simulink model "VirtualADAPT"  (Simulink 8.9)
settings: Solver=ode45  StartTime=0.0  StopTime=inf  RelTol=1e-3  EnableMultiTasking=on
PostLoadFcn:
  | FaultInjectionGUIHandle = FaultInjectionGUI;
InitFcn:
  | VirtualADAPTDataStructure
undefined symbols (not defined in the file or in its folder): ln
libraries: simulink x363
(part 4 of 26)

== system VirtualADAPT/VirtualADAPTv1/PowerDistribution/ACLoadBank1/RelayEY174 ==
  EP2In0 : Inport
  EP1In0 : Inport  Port=2
  SwitchClose : Inport  Port=3
  ParallelConn : Subsystem
      mask Display: image(imread('0.png','BackgroundColor',[1 1 1]))
  Relay : Subsystem
      mask Display: image(imread('1.png','BackgroundColor',[1 1 1]))
  Sensor : Subsystem
  EP2Out0 : Outport
  EP1Out0 : Outport  Port=2
  RelayState : Outport  Port=3
  Current : Outport  Port=4
  Voltage : Outport  Port=5
  wires:
  Relay/1(y(1)) -> ParallelConn/1(u(1))
  ParallelConn/1(y(1)) -> Relay/1(u(1))
  EP2In0/1 -> ParallelConn/2(u(2))
  ParallelConn/2(y(2)) -> EP2Out0/1
  EP1In0/1 -> Relay/2(u(2))
  Relay/2(y(2)) -> EP1Out0/1
  SwitchClose/1 -> Relay/3(u(3)), ParallelConn/3(u(3))
  Relay/3(O) -> Current/1
  ParallelConn/3(O) -> Voltage/1
  Sensor/1(SensedValue) -> RelayState/1
  Relay/4(State) -> Sensor/1(TrueValue)

== system VirtualADAPT/VirtualADAPTv1/PowerDistribution/ACLoadBank1/RelayEY175 ==
  EP2In0 : Inport
  EP1In0 : Inport  Port=2
  SwitchClose : Inport  Port=3
  ParallelConn : Subsystem
      mask Display: image(imread('0.png','BackgroundColor',[1 1 1]))
  Relay : Subsystem
      mask Display: image(imread('1.png','BackgroundColor',[1 1 1]))
  Sensor : Subsystem
  EP2Out0 : Outport
  EP1Out0 : Outport  Port=2
  RelayState : Outport  Port=3
  Current : Outport  Port=4
  Voltage : Outport  Port=5
  wires:
  Relay/1(y(1)) -> ParallelConn/1(u(1))
  ParallelConn/1(y(1)) -> Relay/1(u(1))
  EP2In0/1 -> ParallelConn/2(u(2))
  ParallelConn/2(y(2)) -> EP2Out0/1
  EP1In0/1 -> Relay/2(u(2))
  Relay/2(y(2)) -> EP1Out0/1
  SwitchClose/1 -> Relay/3(u(3)), ParallelConn/3(u(3))
  Relay/3(O) -> Current/1
  ParallelConn/3(O) -> Voltage/1
  Sensor/1(SensedValue) -> RelayState/1
  Relay/4(State) -> Sensor/1(TrueValue)

== system VirtualADAPT/VirtualADAPTv1/PowerDistribution/ACLoadBank2/L2A ==
  VInIn0 : Inport
  PMP1 : Subsystem
  SensorFT520 : Subsystem
  VInOut0 : Outport
  FT520 : Outport  Port=2
  wires:
  VInIn0/1 -> PMP1/1(InputIn0)
  PMP1/1(InputOut0) -> VInOut0/1
  PMP1/3(Flow) -> SensorFT520/1(TrueValue)
  SensorFT520/1(SensedValue) -> FT520/1

== system VirtualADAPT/VirtualADAPTv1/PowerDistribution/ACLoadBank2/L2B ==
  InputIn0 : Inport
  AmbientTemp : Inport  Port=2
  LGT2 : Subsystem
  SensorTE510 : Subsystem
  InputOut0 : Outport
  TE510 : Outport  Port=2
  Current : Outport  Port=3
  wires:
  InputIn0/1 -> LGT2/1(EPIn0)
  LGT2/1(EPOut0) -> InputOut0/1
  LGT2/4(Temperature) -> SensorTE510/1(TrueValue)
  LGT2/3(Current) -> Current/1
  AmbientTemp/1 -> LGT2/2(AmbientTemp)
  SensorTE510/1(SensedValue) -> TE510/1

== system VirtualADAPT/VirtualADAPTv1/PowerDistribution/ACLoadBank2/L2C ==
  EnergyPortIn0 : Inport
  FAN4 : Subsystem
  EnergyPortOut0 : Outport
  wires:
  EnergyPortIn0/1 -> FAN4/1(EPIn0)
  FAN4/1(EPOut0) -> EnergyPortOut0/1

== system VirtualADAPT/VirtualADAPTv1/PowerDistribution/ACLoadBank2/L2D ==
  EnergyPortIn0 : Inport
  LGT9 : Subsystem
  EnergyPortOut0 : Outport
  wires:
  EnergyPortIn0/1 -> LGT9/1(EPIn0)
  LGT9/1(EPOut0) -> EnergyPortOut0/1

== system VirtualADAPT/VirtualADAPTv1/PowerDistribution/ACLoadBank2/L2E ==
  InputIn0 : Inport
  AmbientTemp : Inport  Port=2
  LGT7 : Subsystem
  SensorLT505 : Subsystem
  SensorTE505 : Subsystem
  SensorTE506 : Subsystem
  SensorTE507 : Subsystem
  InputOut0 : Outport
  TE505 : Outport  Port=2
  LT505 : Outport  Port=3
  TE507 : Outport  Port=4
  TE506 : Outport  Port=5
  wires:
  InputIn0/1 -> LGT7/1(VInIn0)
  LGT7/1(VInOut0) -> InputOut0/1
  LGT7/6(Temperature3) -> SensorTE507/1(TrueValue)
  LGT7/4(Temperature1) -> SensorTE505/1(TrueValue)
  AmbientTemp/1 -> LGT7/2(AmbientTemp)
  LGT7/3(Power) -> SensorLT505/1(Power)
  LGT7/5(Temperature2) -> SensorTE506/1(TrueValue)
  SensorTE507/1(SensedValue) -> TE507/1
  SensorTE506/1(SensedValue) -> TE506/1
  SensorTE505/1(SensedValue) -> TE505/1
  SensorLT505/1(Light) -> LT505/1

== system VirtualADAPT/VirtualADAPTv1/PowerDistribution/ACLoadBank2/L2F ==
  VinIn0 : Inport
  FAN2 : Subsystem
  SensorST516 : Subsystem
  VinOut0 : Outport
  ST516 : Outport  Port=2
  Current : Outport  Port=3
  wires:
  VinIn0/1 -> FAN2/1(InputIn0)
  FAN2/1(InputOut0) -> VinOut0/1
  FAN2/3(Current) -> Current/1
  SensorST516/1(SensedValue) -> ST516/1
  FAN2/2(RotationalVelocity) -> SensorST516/1(TrueValue)

== system VirtualADAPT/VirtualADAPTv1/PowerDistribution/ACLoadBank2/RelayEY270 ==
  EP2In0 : Inport
  EP1In0 : Inport  Port=2
  SwitchClose : Inport  Port=3
  ParallelConn : Subsystem
      mask Display: image(imread('0.png','BackgroundColor',[1 1 1]))
  Relay : Subsystem
      mask Display: image(imread('1.png','BackgroundColor',[1 1 1]))
  Sensor : Subsystem
  EP2Out0 : Outport
  EP1Out0 : Outport  Port=2
  RelayState : Outport  Port=3
  Current : Outport  Port=4
  Voltage : Outport  Port=5
  wires:
  Relay/1(y(1)) -> ParallelConn/1(u(1))
  ParallelConn/1(y(1)) -> Relay/1(u(1))
  EP2In0/1 -> ParallelConn/2(u(2))
  ParallelConn/2(y(2)) -> EP2Out0/1
  EP1In0/1 -> Relay/2(u(2))
  Relay/2(y(2)) -> EP1Out0/1
  SwitchClose/1 -> Relay/3(u(3)), ParallelConn/3(u(3))
  Relay/3(O) -> Current/1
  ParallelConn/3(O) -> Voltage/1
  Sensor/1(SensedValue) -> RelayState/1
  Relay/4(State) -> Sensor/1(TrueValue)

== system VirtualADAPT/VirtualADAPTv1/PowerDistribution/ACLoadBank2/RelayEY271 ==
  EP2In0 : Inport
  EP1In0 : Inport  Port=2
  SwitchClose : Inport  Port=3
  ParallelConn : Subsystem
      mask Display: image(imread('0.png','BackgroundColor',[1 1 1]))
  Relay : Subsystem
      mask Display: image(imread('1.png','BackgroundColor',[1 1 1]))
  Sensor : Subsystem
  EP2Out0 : Outport
  EP1Out0 : Outport  Port=2
  RelayState : Outport  Port=3
  Current : Outport  Port=4
  Voltage : Outport  Port=5
  wires:
  Relay/1(y(1)) -> ParallelConn/1(u(1))
  ParallelConn/1(y(1)) -> Relay/1(u(1))
  EP2In0/1 -> ParallelConn/2(u(2))
  ParallelConn/2(y(2)) -> EP2Out0/1
  EP1In0/1 -> Relay/2(u(2))
  Relay/2(y(2)) -> EP1Out0/1
  SwitchClose/1 -> Relay/3(u(3)), ParallelConn/3(u(3))
  Relay/3(O) -> Current/1
  ParallelConn/3(O) -> Voltage/1
  Sensor/1(SensedValue) -> RelayState/1
  Relay/4(State) -> Sensor/1(TrueValue)

== system VirtualADAPT/VirtualADAPTv1/PowerDistribution/ACLoadBank2/RelayEY272 ==
  EP2In0 : Inport
  EP1In0 : Inport  Port=2
  SwitchClose : Inport  Port=3
  ParallelConn : Subsystem
      mask Display: image(imread('0.png','BackgroundColor',[1 1 1]))
  Relay : Subsystem
      mask Display: image(imread('1.png','BackgroundColor',[1 1 1]))
  Sensor : Subsystem
  EP2Out0 : Outport
  EP1Out0 : Outport  Port=2
  RelayState : Outport  Port=3
  Current : Outport  Port=4
  Voltage : Outport  Port=5
  wires:
  Relay/1(y(1)) -> ParallelConn/1(u(1))
  ParallelConn/1(y(1)) -> Relay/1(u(1))
  EP2In0/1 -> ParallelConn/2(u(2))
  ParallelConn/2(y(2)) -> EP2Out0/1
  EP1In0/1 -> Relay/2(u(2))
  Relay/2(y(2)) -> EP1Out0/1
  SwitchClose/1 -> Relay/3(u(3)), ParallelConn/3(u(3))
  Relay/3(O) -> Current/1
  ParallelConn/3(O) -> Voltage/1
  Sensor/1(SensedValue) -> RelayState/1
  Relay/4(State) -> Sensor/1(TrueValue)

== system VirtualADAPT/VirtualADAPTv1/PowerDistribution/ACLoadBank2/RelayEY273 ==
  EP2In0 : Inport
  EP1In0 : Inport  Port=2
  SwitchClose : Inport  Port=3
  ParallelConn : Subsystem
      mask Display: image(imread('0.png','BackgroundColor',[1 1 1]))
  Relay : Subsystem
      mask Display: image(imread('1.png','BackgroundColor',[1 1 1]))
  Sensor : Subsystem
  EP2Out0 : Outport
  EP1Out0 : Outport  Port=2
  RelayState : Outport  Port=3
  Current : Outport  Port=4
  Voltage : Outport  Port=5
  wires:
  Relay/1(y(1)) -> ParallelConn/1(u(1))
  ParallelConn/1(y(1)) -> Relay/1(u(1))
  EP2In0/1 -> ParallelConn/2(u(2))
  ParallelConn/2(y(2)) -> EP2Out0/1
  EP1In0/1 -> Relay/2(u(2))
  Relay/2(y(2)) -> EP1Out0/1
  SwitchClose/1 -> Relay/3(u(3)), ParallelConn/3(u(3))
  Relay/3(O) -> Current/1
  ParallelConn/3(O) -> Voltage/1
  Sensor/1(SensedValue) -> RelayState/1
  Relay/4(State) -> Sensor/1(TrueValue)

== system VirtualADAPT/VirtualADAPTv1/PowerDistribution/ACLoadBank2/RelayEY274 ==
  EP2In0 : Inport
  EP1In0 : Inport  Port=2
  SwitchClose : Inport  Port=3
  ParallelConn : Subsystem
      mask Display: image(imread('0.png','BackgroundColor',[1 1 1]))
  Relay : Subsystem
      mask Display: image(imread('1.png','BackgroundColor',[1 1 1]))
  Sensor : Subsystem
  EP2Out0 : Outport
  EP1Out0 : Outport  Port=2
  RelayState : Outport  Port=3
  Current : Outport  Port=4
  Voltage : Outport  Port=5
  wires:
  Relay/1(y(1)) -> ParallelConn/1(u(1))
  ParallelConn/1(y(1)) -> Relay/1(u(1))
  EP2In0/1 -> ParallelConn/2(u(2))
  ParallelConn/2(y(2)) -> EP2Out0/1
  EP1In0/1 -> Relay/2(u(2))
  Relay/2(y(2)) -> EP1Out0/1
  SwitchClose/1 -> Relay/3(u(3)), ParallelConn/3(u(3))
  Relay/3(O) -> Current/1
  ParallelConn/3(O) -> Voltage/1
  Sensor/1(SensedValue) -> RelayState/1
  Relay/4(State) -> Sensor/1(TrueValue)

== system VirtualADAPT/VirtualADAPTv1/PowerDistribution/ACLoadBank2/RelayEY275 ==
  EP2In0 : Inport
  EP1In0 : Inport  Port=2
  SwitchClose : Inport  Port=3
  ParallelConn : Subsystem
      mask Display: image(imread('0.png','BackgroundColor',[1 1 1]))
  Relay : Subsystem
      mask Display: image(imread('1.png','BackgroundColor',[1 1 1]))
  Sensor : Subsystem
  EP2Out0 : Outport
  EP1Out0 : Outport  Port=2
  RelayState : Outport  Port=3
  Current : Outport  Port=4
  Voltage : Outport  Port=5
  wires:
  Relay/1(y(1)) -> ParallelConn/1(u(1))
  ParallelConn/1(y(1)) -> Relay/1(u(1))
  EP2In0/1 -> ParallelConn/2(u(2))
  ParallelConn/2(y(2)) -> EP2Out0/1
  EP1In0/1 -> Relay/2(u(2))
  Relay/2(y(2)) -> EP1Out0/1
  SwitchClose/1 -> Relay/3(u(3)), ParallelConn/3(u(3))
  Relay/3(O) -> Current/1
  ParallelConn/3(O) -> Voltage/1
  Sensor/1(SensedValue) -> RelayState/1
  Relay/4(State) -> Sensor/1(TrueValue)

== system VirtualADAPT/VirtualADAPTv1/PowerDistribution/CircuitBreakerEY162/CircuitBreakerConnected ==
  Constant : Constant  Value=Constant
  Fault Inject : Subsystem
  Fault Profile : Constant  Value=FP
  Ramp : simulink/Sources/Ramp  slope=FM  start=FT  X0=Constant
  Step : Step  Time=FT  Before=Constant  After=(1 + FM)*Constant  SampleTime=0
  C : Outport
  wires:
  Fault Inject/1(Out1) -> C/1
  Constant/1 -> Fault Inject/1(Nominal)
  Fault Profile/1 -> Fault Inject/2(Fault Profile)
  Step/1 -> Fault Inject/3(Step)
  Ramp/1 -> Fault Inject/4(Ramp)

== system VirtualADAPT/VirtualADAPTv1/PowerDistribution/CircuitBreakerEY162/CurrentLimit ==
  Constant : Constant  Value=Constant
  Fault Inject : Subsystem
  Fault Profile : Constant  Value=FP
  Ramp : simulink/Sources/Ramp  slope=FM  start=FT  X0=Constant
  Step : Step  Time=FT  Before=Constant  After=(1 + FM)*Constant  SampleTime=0
  C : Outport
  wires:
  Fault Inject/1(Out1) -> C/1
  Constant/1 -> Fault Inject/1(Nominal)
  Fault Profile/1 -> Fault Inject/2(Fault Profile)
  Step/1 -> Fault Inject/3(Step)
  Ramp/1 -> Fault Inject/4(Ramp)

== system VirtualADAPT/VirtualADAPTv1/PowerDistribution/CircuitBreakerEY162/CurrentThrough ==
  u(1) : Inport
  u(2) : Inport  Port=2
  u(3) : Inport  Port=3
  u(4) : Inport  Port=4
  Adder : Sum  Inputs=+  SaturateOnIntegerOverflow=on  InputSameDT=on  OutDataTypeStr=Inherit: Same as first input
  Gain : Gain  SaturateOnIntegerOverflow=on  OutDataTypeStr=Inherit: Same as input  ParamDataTypeStr=Inherit: Same as input
  Mux : Mux
  OffGuard : Fcn  Expr=(u(3) || !u(4))
  OnGuard : Fcn  Expr=(0)
  Product1 : Product  SaturateOnIntegerOverflow=on  RndMeth=Zero  InputSameDT=on  OutDataTypeStr=Inherit: Same as first input
  Product2 : Product  SaturateOnIntegerOverflow=on  RndMeth=Zero  InputSameDT=on  OutDataTypeStr=Inherit: Same as first input
  determineState : Subsystem
  y(1) : Outport
  y(2) : Outport  Port=2
  O : Outport  Port=3
  State : Outport  Port=4
  wires:
  Adder/1 -> Gain/1
  u(3)/1 -> Mux/3
  u(4)/1 -> Mux/4
  Mux/1 -> OnGuard/1, OffGuard/1
  OnGuard/1 -> determineState/1(In1)
  OffGuard/1 -> determineState/2(In2)
  u(1)/1 -> Product1/1
  determineState/1(Out1) -> Product1/2, Product2/2, State/1
  Product1/1 -> O/1, y(2)/1
  Gain/1 -> Product2/1
  Product2/1 -> y(1)/1
  u(2)/1 -> Adder/1

== system VirtualADAPT/VirtualADAPTv1/PowerDistribution/CircuitBreakerEY162/DecisionFunction ==
  u(1) : Inport
  u(2) : Inport  Port=2
  Function : Fcn  Expr=u(1)>u(2)
  Mux : Mux  Inputs=2
  One : Constant
  Switch : Switch  Criteria=u2 ~= 0  SaturateOnIntegerOverflow=on  InputSameDT=on
  Zero : Constant  Value=0
  y(1) : Outport
  wires:
  u(1)/1 -> Mux/1
  u(2)/1 -> Mux/2
  Mux/1 -> Function/1
  Function/1 -> Switch/2
  One/1 -> Switch/1
  Zero/1 -> Switch/3
  Switch/1 -> y(1)/1

== system VirtualADAPT/VirtualADAPTv1/PowerDistribution/CircuitBreakerEY162/Delay ==
  Input : Inport
  C : Subsystem  mask{C=0.1, IC=0, FP=Nominal, FT=0, FM=0}
      mask prompts: C "Value of C:"; IC "Initial Condition:"; FP "Fault Profile:"; FT "Fault Injection Time:"; FM "Fault Magnitude/Slope"
      mask TypeOptions FP: Nominal | Abrupt | Incipient
      mask Display: image(imread('C.png','BackgroundColor',[1 1 1]))
  Function : Subsystem
      mask Display: image(imread('fx.png','BackgroundColor',[1 1 1]))
  OneJunction : Subsystem
      mask Display: image(imread('1.png','BackgroundColor',[1 1 1]))
  R : Subsystem  mask{R=1, FP=Nominal, FT=0, FM=0}
      mask prompts: R "Value of R:"; FP "Fault Profile:"; FT "Fault Injection Time:"; FM "Fault Magnitude/Slope"
      mask TypeOptions FP: Nominal | Abrupt | Incipient
      mask Display: image(imread('R.png','BackgroundColor',[1 1 1]))
  Se : Subsystem  mask{FP=Nominal, FT=0, FM=0}
      mask prompts: FP "Fault Profile:"; FT "Fault Injection Time:"; FM "Fault Magnitude/Slope/Bias/StuckAtValue"
      mask TypeOptions FP: Nominal | Abrupt | Incipient | Bias | StuckAt
      mask Display: image(imread('MSe.png','BackgroundColor',[1 1 1]))
  ZeroJunction : Subsystem
      mask Display: image(imread('0.png','BackgroundColor',[1 1 1]))
  Output : Outport
  wires:
  OneJunction/1(y(1)) -> R/1(In)
  R/1(Out) -> OneJunction/1(u(1))
  ZeroJunction/1(y(1)) -> C/1(F)
  C/1(E) -> ZeroJunction/1(u(1))
  OneJunction/2(y(2)) -> ZeroJunction/2(u(2))
  ZeroJunction/2(y(2)) -> OneJunction/2(u(2))
  Se/1(E) -> OneJunction/3(u(3))
  OneJunction/3(y(3)) -> Se/1(F)
  Function/1(y(1)) -> Se/2(Se)
  Input/1 -> Function/1(u(1))
  ZeroJunction/3(O) -> Output/1

== system VirtualADAPT/VirtualADAPTv1/PowerDistribution/CircuitBreakerEY162/ParallelConn ==
  u(1) : Inport
  u(2) : Inport  Port=2
  u(3) : Inport  Port=3
  u(4) : Inport  Port=4
  Adder : Sum  Inputs=-  SaturateOnIntegerOverflow=on  InputSameDT=on  OutDataTypeStr=Inherit: Same as first input
  Gain : Gain  Gain=-1  SaturateOnIntegerOverflow=on  OutDataTypeStr=Inherit: Same as input  ParamDataTypeStr=Inherit: Same as input
  Mux : Mux
  OffGuard : Fcn  Expr=(u(3) || !u(4))
  OnGuard : Fcn  Expr=(0)
  Product1 : Product  SaturateOnIntegerOverflow=on  RndMeth=Zero  InputSameDT=on  OutDataTypeStr=Inherit: Same as first input
  Product2 : Product  SaturateOnIntegerOverflow=on  RndMeth=Zero  InputSameDT=on  OutDataTypeStr=Inherit: Same as first input
  determineState : Subsystem
  y(1) : Outport
  y(2) : Outport  Port=2
  O : Outport  Port=3
  State : Outport  Port=4
  wires:
  Adder/1 -> Gain/1
  u(3)/1 -> Mux/3
  u(4)/1 -> Mux/4
  Mux/1 -> OnGuard/1, OffGuard/1
  OnGuard/1 -> determineState/1(In1)
  OffGuard/1 -> determineState/2(In2)
  u(1)/1 -> Product1/1
  determineState/1(Out1) -> Product1/2, Product2/2, State/1
  Product1/1 -> O/1, y(2)/1
  Gain/1 -> Product2/1
  Product2/1 -> y(1)/1
  u(2)/1 -> Adder/1

== system VirtualADAPT/VirtualADAPTv1/PowerDistribution/CircuitBreakerEY166/CircuitBreakerConnected ==
  Constant : Constant  Value=Constant
  Fault Inject : Subsystem
  Fault Profile : Constant  Value=FP
  Ramp : simulink/Sources/Ramp  slope=FM  start=FT  X0=Constant
  Step : Step  Time=FT  Before=Constant  After=(1 + FM)*Constant  SampleTime=0
  C : Outport
  wires:
  Fault Inject/1(Out1) -> C/1
  Constant/1 -> Fault Inject/1(Nominal)
  Fault Profile/1 -> Fault Inject/2(Fault Profile)
  Step/1 -> Fault Inject/3(Step)
  Ramp/1 -> Fault Inject/4(Ramp)

== system VirtualADAPT/VirtualADAPTv1/PowerDistribution/CircuitBreakerEY166/CurrentLimit ==
  Constant : Constant  Value=Constant
  Fault Inject : Subsystem
  Fault Profile : Constant  Value=FP
  Ramp : simulink/Sources/Ramp  slope=FM  start=FT  X0=Constant
  Step : Step  Time=FT  Before=Constant  After=(1 + FM)*Constant  SampleTime=0
  C : Outport
  wires:
  Fault Inject/1(Out1) -> C/1
  Constant/1 -> Fault Inject/1(Nominal)
  Fault Profile/1 -> Fault Inject/2(Fault Profile)
  Step/1 -> Fault Inject/3(Step)
  Ramp/1 -> Fault Inject/4(Ramp)

== system VirtualADAPT/VirtualADAPTv1/PowerDistribution/CircuitBreakerEY166/CurrentThrough ==
  u(1) : Inport
  u(2) : Inport  Port=2
  u(3) : Inport  Port=3
  u(4) : Inport  Port=4
  Adder : Sum  Inputs=+  SaturateOnIntegerOverflow=on  InputSameDT=on  OutDataTypeStr=Inherit: Same as first input
  Gain : Gain  SaturateOnIntegerOverflow=on  OutDataTypeStr=Inherit: Same as input  ParamDataTypeStr=Inherit: Same as input
  Mux : Mux
  OffGuard : Fcn  Expr=(u(3) || !u(4))
  OnGuard : Fcn  Expr=(0)
  Product1 : Product  SaturateOnIntegerOverflow=on  RndMeth=Zero  InputSameDT=on  OutDataTypeStr=Inherit: Same as first input
  Product2 : Product  SaturateOnIntegerOverflow=on  RndMeth=Zero  InputSameDT=on  OutDataTypeStr=Inherit: Same as first input
  determineState : Subsystem
  y(1) : Outport
  y(2) : Outport  Port=2
  O : Outport  Port=3
  State : Outport  Port=4
  wires:
  Adder/1 -> Gain/1
  u(3)/1 -> Mux/3
  u(4)/1 -> Mux/4
  Mux/1 -> OnGuard/1, OffGuard/1
  OnGuard/1 -> determineState/1(In1)
  OffGuard/1 -> determineState/2(In2)
  u(1)/1 -> Product1/1
  determineState/1(Out1) -> Product1/2, Product2/2, State/1
  Product1/1 -> O/1, y(2)/1
  Gain/1 -> Product2/1
  Product2/1 -> y(1)/1
  u(2)/1 -> Adder/1

== system VirtualADAPT/VirtualADAPTv1/PowerDistribution/CircuitBreakerEY166/DecisionFunction ==
  u(1) : Inport
  u(2) : Inport  Port=2
  Function : Fcn  Expr=u(1)>u(2)
  Mux : Mux  Inputs=2
  One : Constant
  Switch : Switch  Criteria=u2 ~= 0  SaturateOnIntegerOverflow=on  InputSameDT=on
  Zero : Constant  Value=0
  y(1) : Outport
  wires:
  u(1)/1 -> Mux/1
  u(2)/1 -> Mux/2
  Mux/1 -> Function/1
  Function/1 -> Switch/2
  One/1 -> Switch/1
  Zero/1 -> Switch/3
  Switch/1 -> y(1)/1

== system VirtualADAPT/VirtualADAPTv1/PowerDistribution/CircuitBreakerEY166/Delay ==
  Input : Inport
  C : Subsystem  mask{C=0.1, IC=0, FP=Nominal, FT=0, FM=0}
      mask prompts: C "Value of C:"; IC "Initial Condition:"; FP "Fault Profile:"; FT "Fault Injection Time:"; FM "Fault Magnitude/Slope"
      mask TypeOptions FP: Nominal | Abrupt | Incipient
      mask Display: image(imread('C.png','BackgroundColor',[1 1 1]))
  Function : Subsystem
      mask Display: image(imread('fx.png','BackgroundColor',[1 1 1]))
  OneJunction : Subsystem
      mask Display: image(imread('1.png','BackgroundColor',[1 1 1]))
  R : Subsystem  mask{R=1, FP=Nominal, FT=0, FM=0}
      mask prompts: R "Value of R:"; FP "Fault Profile:"; FT "Fault Injection Time:"; FM "Fault Magnitude/Slope"
      mask TypeOptions FP: Nominal | Abrupt | Incipient
      mask Display: image(imread('R.png','BackgroundColor',[1 1 1]))
  Se : Subsystem  mask{FP=Nominal, FT=0, FM=0}
      mask prompts: FP "Fault Profile:"; FT "Fault Injection Time:"; FM "Fault Magnitude/Slope/Bias/StuckAtValue"
      mask TypeOptions FP: Nominal | Abrupt | Incipient | Bias | StuckAt
      mask Display: image(imread('MSe.png','BackgroundColor',[1 1 1]))
  ZeroJunction : Subsystem
      mask Display: image(imread('0.png','BackgroundColor',[1 1 1]))
  Output : Outport
  wires:
  Se/1(E) -> OneJunction/1(u(1))
  OneJunction/1(y(1)) -> Se/1(F)
  OneJunction/2(y(2)) -> ZeroJunction/1(u(1))
  ZeroJunction/1(y(1)) -> OneJunction/2(u(2))
  ZeroJunction/2(y(2)) -> C/1(F)
  C/1(E) -> ZeroJunction/2(u(2))
  OneJunction/3(y(3)) -> R/1(In)
  R/1(Out) -> OneJunction/3(u(3))
  Function/1(y(1)) -> Se/2(Se)
  Input/1 -> Function/1(u(1))
  ZeroJunction/3(O) -> Output/1

== system VirtualADAPT/VirtualADAPTv1/PowerDistribution/CircuitBreakerEY166/ParallelConn ==
  u(1) : Inport
  u(2) : Inport  Port=2
  u(3) : Inport  Port=3
  u(4) : Inport  Port=4
  u(5) : Inport  Port=5
  u(6) : Inport  Port=6
  u(7) : Inport  Port=7
  u(8) : Inport  Port=8
  u(9) : Inport  Port=9
  Adder : Sum  Inputs=------  SaturateOnIntegerOverflow=on  InputSameDT=on  OutDataTypeStr=Inherit: Same as first input
  Gain : Gain  Gain=-1  SaturateOnIntegerOverflow=on  OutDataTypeStr=Inherit: Same as input  ParamDataTypeStr=Inherit: Same as input
  Mux : Mux  Inputs=9
  OffGuard : Fcn  Expr=(u(8) || !u(9))
  OnGuard : Fcn  Expr=(0)
  Product1 : Product  SaturateOnIntegerOverflow=on  RndMeth=Zero  InputSameDT=on  OutDataTypeStr=Inherit: Same as first input
  Product2 : Product  SaturateOnIntegerOverflow=on  RndMeth=Zero  InputSameDT=on  OutDataTypeStr=Inherit: Same as first input
  determineState : Subsystem
  y(1) : Outport
  y(2) : Outport  Port=2
  y(3) : Outport  Port=3
  y(4) : Outport  Port=4
  y(5) : Outport  Port=5
  y(6) : Outport  Port=6
  y(7) : Outport  Port=7
  O : Outport  Port=8
  State : Outport  Port=9
  wires:
  Adder/1 -> Gain/1
  u(8)/1 -> Mux/8
  u(9)/1 -> Mux/9
  Mux/1 -> OnGuard/1, OffGuard/1
  OnGuard/1 -> determineState/1(In1)
  OffGuard/1 -> determineState/2(In2)
  u(1)/1 -> Product1/1
  determineState/1(Out1) -> Product1/2, Product2/2, State/1
  Product1/1 -> O/1, y(2)/1, y(3)/1, y(4)/1, y(5)/1, y(6)/1, y(7)/1
  Gain/1 -> Product2/1
  Product2/1 -> y(1)/1
  u(2)/1 -> Adder/1
  u(3)/1 -> Adder/2
  u(4)/1 -> Adder/3
  u(5)/1 -> Adder/4
  u(6)/1 -> Adder/5
  u(7)/1 -> Adder/6

== system VirtualADAPT/VirtualADAPTv1/PowerDistribution/CircuitBreakerEY180/C ==
  F : Inport
  Capacitance : Constant  Value=C
  Fault Inject : Subsystem
  Fault Profile : Constant  Value=FP
  InitialCondition : Constant  Value=IC
  Integrator : Integrator  InitialConditionSource=external
  Product1 : Product  SaturateOnIntegerOverflow=on  RndMeth=Zero  InputSameDT=on  OutDataTypeStr=Inherit: Same as first input
  Product2 : Product  SaturateOnIntegerOverflow=on  RndMeth=Zero  InputSameDT=on  OutDataTypeStr=Inherit: Same as first input
  Ramp : simulink/Sources/Ramp  slope=FM  start=FT  X0=C
  Reciprocal : Math  Operator=reciprocal  SaturateOnIntegerOverflow=on
  Step : Step  Time=FT  Before=C  After=(1 + FM)*C  SampleTime=0
  E : Outport
  C : Outport  Port=2
  q : Outport  Port=3
  wires:
  F/1 -> Integrator/1
  InitialCondition/1 -> Product1/1
  Capacitance/1 -> Fault Inject/1(Nominal), Product1/2
  Product1/1 -> Integrator/2
  Integrator/1 -> Product2/1, q/1
  Fault Inject/1(Out1) -> Reciprocal/1, C/1
  Reciprocal/1 -> Product2/2
  Fault Profile/1 -> Fault Inject/2(Fault Profile)
  Step/1 -> Fault Inject/3(Step)
  Ramp/1 -> Fault Inject/4(Ramp)
  Product2/1 -> E/1

== system VirtualADAPT/VirtualADAPTv1/PowerDistribution/CircuitBreakerEY180/CircuitBreakerConnected ==
  Constant : Constant  Value=Constant
  Fault Inject : Subsystem
  Fault Profile : Constant  Value=FP
  Ramp : simulink/Sources/Ramp  slope=FM  start=FT  X0=Constant
  Step : Step  Time=FT  Before=Constant  After=(1 + FM)*Constant  SampleTime=0
  C : Outport
  wires:
  Fault Inject/1(Out1) -> C/1
  Constant/1 -> Fault Inject/1(Nominal)
  Fault Profile/1 -> Fault Inject/2(Fault Profile)
  Step/1 -> Fault Inject/3(Step)
  Ramp/1 -> Fault Inject/4(Ramp)

== system VirtualADAPT/VirtualADAPTv1/PowerDistribution/CircuitBreakerEY180/CurrentLimit ==
  Constant : Constant  Value=Constant
  Fault Inject : Subsystem
  Fault Profile : Constant  Value=FP
  Ramp : simulink/Sources/Ramp  slope=FM  start=FT  X0=Constant
  Step : Step  Time=FT  Before=Constant  After=(1 + FM)*Constant  SampleTime=0
  C : Outport
  wires:
  Fault Inject/1(Out1) -> C/1
  Constant/1 -> Fault Inject/1(Nominal)
  Fault Profile/1 -> Fault Inject/2(Fault Profile)
  Step/1 -> Fault Inject/3(Step)
  Ramp/1 -> Fault Inject/4(Ramp)

== system VirtualADAPT/VirtualADAPTv1/PowerDistribution/CircuitBreakerEY180/CurrentThrough ==
  u(1) : Inport
  u(2) : Inport  Port=2
  u(3) : Inport  Port=3
  u(4) : Inport  Port=4
  u(5) : Inport  Port=5
  Adder : Sum  Inputs=-+  SaturateOnIntegerOverflow=on  InputSameDT=on  OutDataTypeStr=Inherit: Same as first input
  Gain : Gain  SaturateOnIntegerOverflow=on  OutDataTypeStr=Inherit: Same as input  ParamDataTypeStr=Inherit: Same as input
  Mux : Mux  Inputs=5
  OffGuard : Fcn  Expr=(u(4) || !u(5))
  OnGuard : Fcn  Expr=(0)
  Product1 : Product  SaturateOnIntegerOverflow=on  RndMeth=Zero  InputSameDT=on  OutDataTypeStr=Inherit: Same as first input
  Product2 : Product  SaturateOnIntegerOverflow=on  RndMeth=Zero  InputSameDT=on  OutDataTypeStr=Inherit: Same as first input
  determineState : Subsystem
  y(1) : Outport
  y(2) : Outport  Port=2
  y(3) : Outport  Port=3
  O : Outport  Port=4
  State : Outport  Port=5
  wires:
  Adder/1 -> Gain/1
  u(4)/1 -> Mux/4
  u(5)/1 -> Mux/5
  Mux/1 -> OnGuard/1, OffGuard/1
  OnGuard/1 -> determineState/1(In1)
  OffGuard/1 -> determineState/2(In2)
  u(2)/1 -> Product1/1
  determineState/1(Out1) -> Product1/2, Product2/2, State/1
  Product1/1 -> O/1, y(1)/1, y(3)/1
  Gain/1 -> Product2/1
  u(1)/1 -> Adder/1
  Product2/1 -> y(2)/1
  u(3)/1 -> Adder/2

== system VirtualADAPT/VirtualADAPTv1/PowerDistribution/CircuitBreakerEY180/DecisionFunction ==
  u(1) : Inport
  u(2) : Inport  Port=2
  Function : Fcn  Expr=u(2)>u(1)
  Mux : Mux  Inputs=2
  One : Constant
  Switch : Switch  Criteria=u2 ~= 0  SaturateOnIntegerOverflow=on  InputSameDT=on
  Zero : Constant  Value=0
  y(1) : Outport
  wires:
  u(1)/1 -> Mux/1
  u(2)/1 -> Mux/2
  Mux/1 -> Function/1
  Function/1 -> Switch/2
  One/1 -> Switch/1
  Zero/1 -> Switch/3
  Switch/1 -> y(1)/1

== system VirtualADAPT/VirtualADAPTv1/PowerDistribution/CircuitBreakerEY180/Delay ==
  Input : Inport
  C : Subsystem  mask{C=0.8, IC=0, FP=Nominal, FT=0, FM=0}
      mask prompts: C "Value of C:"; IC "Initial Condition:"; FP "Fault Profile:"; FT "Fault Injection Time:"; FM "Fault Magnitude/Slope"
      mask TypeOptions FP: Nominal | Abrupt | Incipient
      mask Display: image(imread('C.png','BackgroundColor',[1 1 1]))
  Function : Subsystem
      mask Display: image(imread('fx.png','BackgroundColor',[1 1 1]))
  OneJunction : Subsystem
      mask Display: image(imread('1.png','BackgroundColor',[1 1 1]))
  R : Subsystem  mask{R=1, FP=Nominal, FT=0, FM=0}
      mask prompts: R "Value of R:"; FP "Fault Profile:"; FT "Fault Injection Time:"; FM "Fault Magnitude/Slope"
      mask TypeOptions FP: Nominal | Abrupt | Incipient
      mask Display: image(imread('R.png','BackgroundColor',[1 1 1]))
  Se : Subsystem  mask{FP=Nominal, FT=0, FM=0}
      mask prompts: FP "Fault Profile:"; FT "Fault Injection Time:"; FM "Fault Magnitude/Slope/Bias/StuckAtValue"
      mask TypeOptions FP: Nominal | Abrupt | Incipient | Bias | StuckAt
      mask Display: image(imread('MSe.png','BackgroundColor',[1 1 1]))
  ZeroJunction : Subsystem
      mask Display: image(imread('0.png','BackgroundColor',[1 1 1]))
  Output : Outport
  wires:
  Se/1(E) -> OneJunction/1(u(1))
  OneJunction/1(y(1)) -> Se/1(F)
  OneJunction/2(y(2)) -> ZeroJunction/1(u(1))
  ZeroJunction/1(y(1)) -> OneJunction/2(u(2))
  OneJunction/3(y(3)) -> R/1(In)
  R/1(Out) -> OneJunction/3(u(3))
  ZeroJunction/2(y(2)) -> C/1(F)
  C/1(E) -> ZeroJunction/2(u(2))
  Function/1(y(1)) -> Se/2(Se)
  Input/1 -> Function/1(u(1))
  ZeroJunction/3(O) -> Output/1

== system VirtualADAPT/VirtualADAPTv1/PowerDistribution/CircuitBreakerEY180/ParallelConn ==
  u(1) : Inport
  u(2) : Inport  Port=2
  u(3) : Inport  Port=3
  u(4) : Inport  Port=4
  u(5) : Inport  Port=5
  Adder : Sum  Inputs=--  SaturateOnIntegerOverflow=on  InputSameDT=on  OutDataTypeStr=Inherit: Same as first input
  Gain : Gain  Gain=-1  SaturateOnIntegerOverflow=on  OutDataTypeStr=Inherit: Same as input  ParamDataTypeStr=Inherit: Same as input
  Mux : Mux  Inputs=5
  OffGuard : Fcn  Expr=(u(4) || !u(5))
  OnGuard : Fcn  Expr=(0)
  Product1 : Product  SaturateOnIntegerOverflow=on  RndMeth=Zero  InputSameDT=on  OutDataTypeStr=Inherit: Same as first input
  Product2 : Product  SaturateOnIntegerOverflow=on  RndMeth=Zero  InputSameDT=on  OutDataTypeStr=Inherit: Same as first input
  determineState : Subsystem
  y(1) : Outport
  y(2) : Outport  Port=2
  y(3) : Outport  Port=3
  O : Outport  Port=4
  State : Outport  Port=5
  wires:
  Adder/1 -> Gain/1
  u(4)/1 -> Mux/4
  u(5)/1 -> Mux/5
  Mux/1 -> OnGuard/1, OffGuard/1
  OnGuard/1 -> determineState/1(In1)
  OffGuard/1 -> determineState/2(In2)
  u(1)/1 -> Product1/1
  determineState/1(Out1) -> Product1/2, Product2/2, State/1
  Product1/1 -> O/1, y(2)/1, y(3)/1
  Gain/1 -> Product2/1
  Product2/1 -> y(1)/1
  u(2)/1 -> Adder/1
  u(3)/1 -> Adder/2

== system VirtualADAPT/VirtualADAPTv1/PowerDistribution/CircuitBreakerEY180/R ==
  In : Inport
  Bond : Constant  Value=2
  Computing R : Subsystem
  Fault Profile : Constant  Value=FP
  JuncDB : Constant  Value=3
  Junction Type : Constant  Value=0
  Ramp : simulink/Sources/Ramp  slope=FM  start=FT  X0=R
  Resistor Value : Constant  Value=R
  Step : Step  Time=FT  Before=R  After=(1 + FM)*R  SampleTime=0
  Out : Outport
  R : Outport  Port=2
  wires:
  Computing R/1(Out1) -> Out/1
  In/1 -> Computing R/1(In1)
  Resistor Value/1 -> Computing R/2(RNominal)
  Fault Profile/1 -> Computing R/3(Fault Profile)
  Step/1 -> Computing R/4(Step)
  Ramp/1 -> Computing R/5(Ramp)
  JuncDB/1 -> Computing R/6(JuncDB)
  Bond/1 -> Computing R/7(Bond)
  Junction Type/1 -> Computing R/8(Junction Type)
  Computing R/2(Out2) -> R/1

== system VirtualADAPT/VirtualADAPTv1/PowerDistribution/CircuitBreakerEY180/ZeroJunc ==
  u(1) : Inport
  u(2) : Inport  Port=2
  u(3) : Inport  Port=3
  Adder : Sum  Inputs=+-  SaturateOnIntegerOverflow=on  InputSameDT=on  OutDataTypeStr=Inherit: Same as first input
  Gain : Gain  SaturateOnIntegerOverflow=on  OutDataTypeStr=Inherit: Same as input  ParamDataTypeStr=Inherit: Same as input
  y(1) : Outport
  y(2) : Outport  Port=2
  y(3) : Outport  Port=3
  O : Outport  Port=4
  wires:
  Adder/1 -> Gain/1
  u(3)/1 -> y(1)/1, y(2)/1, O/1
  u(1)/1 -> Adder/1
  u(2)/1 -> Adder/2
  Gain/1 -> y(3)/1

== system VirtualADAPT/VirtualADAPTv1/PowerDistribution/CircuitBreakerEY262/CircuitBreakerConnected ==
  Constant : Constant  Value=Constant
  Fault Inject : Subsystem
  Fault Profile : Constant  Value=FP
  Ramp : simulink/Sources/Ramp  slope=FM  start=FT  X0=Constant
  Step : Step  Time=FT  Before=Constant  After=(1 + FM)*Constant  SampleTime=0
  C : Outport
  wires:
  Fault Inject/1(Out1) -> C/1
  Constant/1 -> Fault Inject/1(Nominal)
  Fault Profile/1 -> Fault Inject/2(Fault Profile)
  Step/1 -> Fault Inject/3(Step)
  Ramp/1 -> Fault Inject/4(Ramp)

== system VirtualADAPT/VirtualADAPTv1/PowerDistribution/CircuitBreakerEY262/CurrentLimit ==
  Constant : Constant  Value=Constant
  Fault Inject : Subsystem
  Fault Profile : Constant  Value=FP
  Ramp : simulink/Sources/Ramp  slope=FM  start=FT  X0=Constant
  Step : Step  Time=FT  Before=Constant  After=(1 + FM)*Constant  SampleTime=0
  C : Outport
  wires:
  Fault Inject/1(Out1) -> C/1
  Constant/1 -> Fault Inject/1(Nominal)
  Fault Profile/1 -> Fault Inject/2(Fault Profile)
  Step/1 -> Fault Inject/3(Step)
  Ramp/1 -> Fault Inject/4(Ramp)

== system VirtualADAPT/VirtualADAPTv1/PowerDistribution/CircuitBreakerEY262/CurrentThrough ==
  u(1) : Inport
  u(2) : Inport  Port=2
  u(3) : Inport  Port=3
  u(4) : Inport  Port=4
  Adder : Sum  Inputs=+  SaturateOnIntegerOverflow=on  InputSameDT=on  OutDataTypeStr=Inherit: Same as first input
  Gain : Gain  SaturateOnIntegerOverflow=on  OutDataTypeStr=Inherit: Same as input  ParamDataTypeStr=Inherit: Same as input
  Mux : Mux
  OffGuard : Fcn  Expr=(u(3) || !u(4))
  OnGuard : Fcn  Expr=(0)
  Product1 : Product  SaturateOnIntegerOverflow=on  RndMeth=Zero  InputSameDT=on  OutDataTypeStr=Inherit: Same as first input
  Product2 : Product  SaturateOnIntegerOverflow=on  RndMeth=Zero  InputSameDT=on  OutDataTypeStr=Inherit: Same as first input
  determineState : Subsystem
  y(1) : Outport
  y(2) : Outport  Port=2
  O : Outport  Port=3
  State : Outport  Port=4
  wires:
  Adder/1 -> Gain/1
  u(3)/1 -> Mux/3
  u(4)/1 -> Mux/4
  Mux/1 -> OnGuard/1, OffGuard/1
  OnGuard/1 -> determineState/1(In1)
  OffGuard/1 -> determineState/2(In2)
  u(1)/1 -> Product1/1
  determineState/1(Out1) -> Product1/2, Product2/2, State/1
  Product1/1 -> O/1, y(2)/1
  Gain/1 -> Product2/1
  Product2/1 -> y(1)/1
  u(2)/1 -> Adder/1

== system VirtualADAPT/VirtualADAPTv1/PowerDistribution/CircuitBreakerEY262/DecisionFunction ==
  u(1) : Inport
  u(2) : Inport  Port=2
  Function : Fcn  Expr=u(2)>u(1)
  Mux : Mux  Inputs=2
  One : Constant
  Switch : Switch  Criteria=u2 ~= 0  SaturateOnIntegerOverflow=on  InputSameDT=on
  Zero : Constant  Value=0
  y(1) : Outport
  wires:
  u(1)/1 -> Mux/1
  u(2)/1 -> Mux/2
  Mux/1 -> Function/1
  Function/1 -> Switch/2
  One/1 -> Switch/1
  Zero/1 -> Switch/3
  Switch/1 -> y(1)/1

== system VirtualADAPT/VirtualADAPTv1/PowerDistribution/CircuitBreakerEY262/Delay ==
  Input : Inport
  C : Subsystem  mask{C=0.1, IC=0, FP=Nominal, FT=0, FM=0}
      mask prompts: C "Value of C:"; IC "Initial Condition:"; FP "Fault Profile:"; FT "Fault Injection Time:"; FM "Fault Magnitude/Slope"
      mask TypeOptions FP: Nominal | Abrupt | Incipient
      mask Display: image(imread('C.png','BackgroundColor',[1 1 1]))
  Function : Subsystem
      mask Display: image(imread('fx.png','BackgroundColor',[1 1 1]))
  OneJunction : Subsystem
      mask Display: image(imread('1.png','BackgroundColor',[1 1 1]))
  R : Subsystem  mask{R=1, FP=Nominal, FT=0, FM=0}
      mask prompts: R "Value of R:"; FP "Fault Profile:"; FT "Fault Injection Time:"; FM "Fault Magnitude/Slope"
      mask TypeOptions FP: Nominal | Abrupt | Incipient
      mask Display: image(imread('R.png','BackgroundColor',[1 1 1]))
  Se : Subsystem  mask{FP=Nominal, FT=0, FM=0}
      mask prompts: FP "Fault Profile:"; FT "Fault Injection Time:"; FM "Fault Magnitude/Slope/Bias/StuckAtValue"
      mask TypeOptions FP: Nominal | Abrupt | Incipient | Bias | StuckAt
      mask Display: image(imread('MSe.png','BackgroundColor',[1 1 1]))
  ZeroJunction : Subsystem
      mask Display: image(imread('0.png','BackgroundColor',[1 1 1]))
  Output : Outport
  wires:
  OneJunction/1(y(1)) -> ZeroJunction/1(u(1))
  ZeroJunction/1(y(1)) -> OneJunction/1(u(1))
  OneJunction/2(y(2)) -> R/1(In)
  R/1(Out) -> OneJunction/2(u(2))
  ZeroJunction/2(y(2)) -> C/1(F)
  C/1(E) -> ZeroJunction/2(u(2))
  Se/1(E) -> OneJunction/3(u(3))
  OneJunction/3(y(3)) -> Se/1(F)
  Function/1(y(1)) -> Se/2(Se)
  Input/1 -> Function/1(u(1))
  ZeroJunction/3(O) -> Output/1

== system VirtualADAPT/VirtualADAPTv1/PowerDistribution/CircuitBreakerEY262/ParallelConn ==
  u(1) : Inport
  u(2) : Inport  Port=2
  u(3) : Inport  Port=3
  u(4) : Inport  Port=4
  Adder : Sum  Inputs=-  SaturateOnIntegerOverflow=on  InputSameDT=on  OutDataTypeStr=Inherit: Same as first input
  Gain : Gain  Gain=-1  SaturateOnIntegerOverflow=on  OutDataTypeStr=Inherit: Same as input  ParamDataTypeStr=Inherit: Same as input
  Mux : Mux
  OffGuard : Fcn  Expr=(u(3) || !u(4))
  OnGuard : Fcn  Expr=(0)
  Product1 : Product  SaturateOnIntegerOverflow=on  RndMeth=Zero  InputSameDT=on  OutDataTypeStr=Inherit: Same as first input
  Product2 : Product  SaturateOnIntegerOverflow=on  RndMeth=Zero  InputSameDT=on  OutDataTypeStr=Inherit: Same as first input
  determineState : Subsystem
  y(1) : Outport
  y(2) : Outport  Port=2
  O : Outport  Port=3
  State : Outport  Port=4
  wires:
  Adder/1 -> Gain/1
  u(3)/1 -> Mux/3
  u(4)/1 -> Mux/4
  Mux/1 -> OnGuard/1, OffGuard/1
  OnGuard/1 -> determineState/1(In1)
  OffGuard/1 -> determineState/2(In2)
  u(1)/1 -> Product1/1
  determineState/1(Out1) -> Product1/2, Product2/2, State/1
  Product1/1 -> O/1, y(2)/1
  Gain/1 -> Product2/1
  Product2/1 -> y(1)/1
  u(2)/1 -> Adder/1

== system VirtualADAPT/VirtualADAPTv1/PowerDistribution/CircuitBreakerEY266/CircuitBreakerConnected ==
  Constant : Constant  Value=Constant
  Fault Inject : Subsystem
  Fault Profile : Constant  Value=FP
  Ramp : simulink/Sources/Ramp  slope=FM  start=FT  X0=Constant
  Step : Step  Time=FT  Before=Constant  After=(1 + FM)*Constant  SampleTime=0
  C : Outport
  wires:
  Fault Inject/1(Out1) -> C/1
  Constant/1 -> Fault Inject/1(Nominal)
  Fault Profile/1 -> Fault Inject/2(Fault Profile)
  Step/1 -> Fault Inject/3(Step)
  Ramp/1 -> Fault Inject/4(Ramp)

== system VirtualADAPT/VirtualADAPTv1/PowerDistribution/CircuitBreakerEY266/CurrentLimit ==
  Constant : Constant  Value=Constant
  Fault Inject : Subsystem
  Fault Profile : Constant  Value=FP
  Ramp : simulink/Sources/Ramp  slope=FM  start=FT  X0=Constant
  Step : Step  Time=FT  Before=Constant  After=(1 + FM)*Constant  SampleTime=0
  C : Outport
  wires:
  Fault Inject/1(Out1) -> C/1
  Constant/1 -> Fault Inject/1(Nominal)
  Fault Profile/1 -> Fault Inject/2(Fault Profile)
  Step/1 -> Fault Inject/3(Step)
  Ramp/1 -> Fault Inject/4(Ramp)

== system VirtualADAPT/VirtualADAPTv1/PowerDistribution/CircuitBreakerEY266/CurrentThrough ==
  u(1) : Inport
  u(2) : Inport  Port=2
  u(3) : Inport  Port=3
  u(4) : Inport  Port=4
  Adder : Sum  Inputs=+  SaturateOnIntegerOverflow=on  InputSameDT=on  OutDataTypeStr=Inherit: Same as first input
  Gain : Gain  SaturateOnIntegerOverflow=on  OutDataTypeStr=Inherit: Same as input  ParamDataTypeStr=Inherit: Same as input
  Mux : Mux
  OffGuard : Fcn  Expr=(u(3) || !u(4))
  OnGuard : Fcn  Expr=(0)
  Product1 : Product  SaturateOnIntegerOverflow=on  RndMeth=Zero  InputSameDT=on  OutDataTypeStr=Inherit: Same as first input
  Product2 : Product  SaturateOnIntegerOverflow=on  RndMeth=Zero  InputSameDT=on  OutDataTypeStr=Inherit: Same as first input
  determineState : Subsystem
  y(1) : Outport
  y(2) : Outport  Port=2
  O : Outport  Port=3
  State : Outport  Port=4
  wires:
  Adder/1 -> Gain/1
  u(3)/1 -> Mux/3
  u(4)/1 -> Mux/4
  Mux/1 -> OnGuard/1, OffGuard/1
  OnGuard/1 -> determineState/1(In1)
  OffGuard/1 -> determineState/2(In2)
  u(1)/1 -> Product1/1
  determineState/1(Out1) -> Product1/2, Product2/2, State/1
  Product1/1 -> O/1, y(2)/1
  Gain/1 -> Product2/1
  Product2/1 -> y(1)/1
  u(2)/1 -> Adder/1

== system VirtualADAPT/VirtualADAPTv1/PowerDistribution/CircuitBreakerEY266/DecisionFunction ==
  u(1) : Inport
  u(2) : Inport  Port=2
  Function : Fcn  Expr=u(1)>u(2)
  Mux : Mux  Inputs=2
  One : Constant
  Switch : Switch  Criteria=u2 ~= 0  SaturateOnIntegerOverflow=on  InputSameDT=on
  Zero : Constant  Value=0
  y(1) : Outport
  wires:
  u(1)/1 -> Mux/1
  u(2)/1 -> Mux/2
  Mux/1 -> Function/1
  Function/1 -> Switch/2
  One/1 -> Switch/1
  Zero/1 -> Switch/3
  Switch/1 -> y(1)/1

== system VirtualADAPT/VirtualADAPTv1/PowerDistribution/CircuitBreakerEY266/Delay ==
  Input : Inport
  C : Subsystem  mask{C=0.1, IC=0, FP=Nominal, FT=0, FM=0}
      mask prompts: C "Value of C:"; IC "Initial Condition:"; FP "Fault Profile:"; FT "Fault Injection Time:"; FM "Fault Magnitude/Slope"
      mask TypeOptions FP: Nominal | Abrupt | Incipient
      mask Display: image(imread('C.png','BackgroundColor',[1 1 1]))
  Function : Subsystem
      mask Display: image(imread('fx.png','BackgroundColor',[1 1 1]))
  OneJunction : Subsystem
      mask Display: image(imread('1.png','BackgroundColor',[1 1 1]))
  R : Subsystem  mask{R=1, FP=Nominal, FT=0, FM=0}
      mask prompts: R "Value of R:"; FP "Fault Profile:"; FT "Fault Injection Time:"; FM "Fault Magnitude/Slope"
      mask TypeOptions FP: Nominal | Abrupt | Incipient
      mask Display: image(imread('R.png','BackgroundColor',[1 1 1]))
  Se : Subsystem  mask{FP=Nominal, FT=0, FM=0}
      mask prompts: FP "Fault Profile:"; FT "Fault Injection Time:"; FM "Fault Magnitude/Slope/Bias/StuckAtValue"
      mask TypeOptions FP: Nominal | Abrupt | Incipient | Bias | StuckAt
      mask Display: image(imread('MSe.png','BackgroundColor',[1 1 1]))
  ZeroJunction : Subsystem
      mask Display: image(imread('0.png','BackgroundColor',[1 1 1]))
  Output : Outport
  wires:
  Se/1(E) -> OneJunction/1(u(1))
  OneJunction/1(y(1)) -> Se/1(F)
  OneJunction/2(y(2)) -> ZeroJunction/1(u(1))
  ZeroJunction/1(y(1)) -> OneJunction/2(u(2))
  ZeroJunction/2(y(2)) -> C/1(F)
  C/1(E) -> ZeroJunction/2(u(2))
  OneJunction/3(y(3)) -> R/1(In)
  R/1(Out) -> OneJunction/3(u(3))
  Function/1(y(1)) -> Se/2(Se)
  Input/1 -> Function/1(u(1))
  ZeroJunction/3(O) -> Output/1

== system VirtualADAPT/VirtualADAPTv1/PowerDistribution/CircuitBreakerEY266/ParallelConn ==
  u(1) : Inport
  u(2) : Inport  Port=2
  u(3) : Inport  Port=3
  u(4) : Inport  Port=4
  u(5) : Inport  Port=5
  u(6) : Inport  Port=6
  u(7) : Inport  Port=7
  u(8) : Inport  Port=8
  u(9) : Inport  Port=9
  Adder : Sum  Inputs=------  SaturateOnIntegerOverflow=on  InputSameDT=on  OutDataTypeStr=Inherit: Same as first input
  Gain : Gain  Gain=-1  SaturateOnIntegerOverflow=on  OutDataTypeStr=Inherit: Same as input  ParamDataTypeStr=Inherit: Same as input
  Mux : Mux  Inputs=9
  OffGuard : Fcn  Expr=(u(8) || !u(9))
  OnGuard : Fcn  Expr=(0)
  Product1 : Product  SaturateOnIntegerOverflow=on  RndMeth=Zero  InputSameDT=on  OutDataTypeStr=Inherit: Same as first input
  Product2 : Product  SaturateOnIntegerOverflow=on  RndMeth=Zero  InputSameDT=on  OutDataTypeStr=Inherit: Same as first input
  determineState : Subsystem
  y(1) : Outport
  y(2) : Outport  Port=2
  y(3) : Outport  Port=3
  y(4) : Outport  Port=4
  y(5) : Outport  Port=5
  y(6) : Outport  Port=6
  y(7) : Outport  Port=7
  O : Outport  Port=8
  State : Outport  Port=9
  wires:
  Adder/1 -> Gain/1
  u(8)/1 -> Mux/8
  u(9)/1 -> Mux/9
  Mux/1 -> OnGuard/1, OffGuard/1
  OnGuard/1 -> determineState/1(In1)
  OffGuard/1 -> determineState/2(In2)
  u(1)/1 -> Product1/1
  determineState/1(Out1) -> Product1/2, Product2/2, State/1
  Product1/1 -> O/1, y(2)/1, y(3)/1, y(4)/1, y(5)/1, y(6)/1, y(7)/1
  Gain/1 -> Product2/1
  Product2/1 -> y(1)/1
  u(2)/1 -> Adder/1
  u(3)/1 -> Adder/2
  u(4)/1 -> Adder/3
  u(5)/1 -> Adder/4
  u(6)/1 -> Adder/5
  u(7)/1 -> Adder/6

== system VirtualADAPT/VirtualADAPTv1/PowerDistribution/CircuitBreakerEY280/C ==
  F : Inport
  Capacitance : Constant  Value=C
  Fault Inject : Subsystem
  Fault Profile : Constant  Value=FP
  InitialCondition : Constant  Value=IC
  Integrator : Integrator  InitialConditionSource=external
  Product1 : Product  SaturateOnIntegerOverflow=on  RndMeth=Zero  InputSameDT=on  OutDataTypeStr=Inherit: Same as first input
  Product2 : Product  SaturateOnIntegerOverflow=on  RndMeth=Zero  InputSameDT=on  OutDataTypeStr=Inherit: Same as first input
  Ramp : simulink/Sources/Ramp  slope=FM  start=FT  X0=C
  Reciprocal : Math  Operator=reciprocal  SaturateOnIntegerOverflow=on
  Step : Step  Time=FT  Before=C  After=(1 + FM)*C  SampleTime=0
  E : Outport
  C : Outport  Port=2
  q : Outport  Port=3
  wires:
  F/1 -> Integrator/1
  InitialCondition/1 -> Product1/1
  Capacitance/1 -> Fault Inject/1(Nominal), Product1/2
  Product1/1 -> Integrator/2
  Integrator/1 -> Product2/1, q/1
  Fault Inject/1(Out1) -> Reciprocal/1, C/1
  Reciprocal/1 -> Product2/2
  Fault Profile/1 -> Fault Inject/2(Fault Profile)
  Step/1 -> Fault Inject/3(Step)
  Ramp/1 -> Fault Inject/4(Ramp)
  Product2/1 -> E/1

== system VirtualADAPT/VirtualADAPTv1/PowerDistribution/CircuitBreakerEY280/CircuitBreakerConnected ==
  Constant : Constant  Value=Constant
  Fault Inject : Subsystem
  Fault Profile : Constant  Value=FP
  Ramp : simulink/Sources/Ramp  slope=FM  start=FT  X0=Constant
  Step : Step  Time=FT  Before=Constant  After=(1 + FM)*Constant  SampleTime=0
  C : Outport
  wires:
  Fault Inject/1(Out1) -> C/1
  Constant/1 -> Fault Inject/1(Nominal)
  Fault Profile/1 -> Fault Inject/2(Fault Profile)
  Step/1 -> Fault Inject/3(Step)
  Ramp/1 -> Fault Inject/4(Ramp)

== system VirtualADAPT/VirtualADAPTv1/PowerDistribution/CircuitBreakerEY280/CurrentLimit ==
  Constant : Constant  Value=Constant
  Fault Inject : Subsystem
  Fault Profile : Constant  Value=FP
  Ramp : simulink/Sources/Ramp  slope=FM  start=FT  X0=Constant
  Step : Step  Time=FT  Before=Constant  After=(1 + FM)*Constant  SampleTime=0
  C : Outport
  wires:
  Fault Inject/1(Out1) -> C/1
  Constant/1 -> Fault Inject/1(Nominal)
  Fault Profile/1 -> Fault Inject/2(Fault Profile)
  Step/1 -> Fault Inject/3(Step)
  Ramp/1 -> Fault Inject/4(Ramp)

== system VirtualADAPT/VirtualADAPTv1/PowerDistribution/CircuitBreakerEY280/CurrentThrough ==
  u(1) : Inport
  u(2) : Inport  Port=2
  u(3) : Inport  Port=3
  u(4) : Inport  Port=4
  u(5) : Inport  Port=5
  Adder : Sum  Inputs=-+  SaturateOnIntegerOverflow=on  InputSameDT=on  OutDataTypeStr=Inherit: Same as first input
  Gain : Gain  SaturateOnIntegerOverflow=on  OutDataTypeStr=Inherit: Same as input  ParamDataTypeStr=Inherit: Same as input
  Mux : Mux  Inputs=5
  OffGuard : Fcn  Expr=(u(4) || !u(5))
  OnGuard : Fcn  Expr=(0)
  Product1 : Product  SaturateOnIntegerOverflow=on  RndMeth=Zero  InputSameDT=on  OutDataTypeStr=Inherit: Same as first input
  Product2 : Product  SaturateOnIntegerOverflow=on  RndMeth=Zero  InputSameDT=on  OutDataTypeStr=Inherit: Same as first input
  determineState : Subsystem
  y(1) : Outport
  y(2) : Outport  Port=2
  y(3) : Outport  Port=3
  O : Outport  Port=4
  State : Outport  Port=5
  wires:
  Adder/1 -> Gain/1
  u(4)/1 -> Mux/4
  u(5)/1 -> Mux/5
  Mux/1 -> OnGuard/1, OffGuard/1
  OnGuard/1 -> determineState/1(In1)
  OffGuard/1 -> determineState/2(In2)
  u(2)/1 -> Product1/1
  determineState/1(Out1) -> Product1/2, Product2/2, State/1
  Product1/1 -> O/1, y(1)/1, y(3)/1
  Gain/1 -> Product2/1
  u(1)/1 -> Adder/1
  Product2/1 -> y(2)/1
  u(3)/1 -> Adder/2

== system VirtualADAPT/VirtualADAPTv1/PowerDistribution/CircuitBreakerEY280/DecisionFunction ==
  u(1) : Inport
  u(2) : Inport  Port=2
  Function : Fcn  Expr=u(2)>u(1)
  Mux : Mux  Inputs=2
  One : Constant
  Switch : Switch  Criteria=u2 ~= 0  SaturateOnIntegerOverflow=on  InputSameDT=on
  Zero : Constant  Value=0
  y(1) : Outport
  wires:
  u(1)/1 -> Mux/1
  u(2)/1 -> Mux/2
  Mux/1 -> Function/1
  Function/1 -> Switch/2
  One/1 -> Switch/1
  Zero/1 -> Switch/3
  Switch/1 -> y(1)/1

== system VirtualADAPT/VirtualADAPTv1/PowerDistribution/CircuitBreakerEY280/Delay ==
  Input : Inport
  C : Subsystem  mask{C=0.8, IC=0, FP=Nominal, FT=0, FM=0}
      mask prompts: C "Value of C:"; IC "Initial Condition:"; FP "Fault Profile:"; FT "Fault Injection Time:"; FM "Fault Magnitude/Slope"
      mask TypeOptions FP: Nominal | Abrupt | Incipient
      mask Display: image(imread('C.png','BackgroundColor',[1 1 1]))
  Function : Subsystem
      mask Display: image(imread('fx.png','BackgroundColor',[1 1 1]))
  OneJunction : Subsystem
      mask Display: image(imread('1.png','BackgroundColor',[1 1 1]))
  R : Subsystem  mask{R=1, FP=Nominal, FT=0, FM=0}
      mask prompts: R "Value of R:"; FP "Fault Profile:"; FT "Fault Injection Time:"; FM "Fault Magnitude/Slope"
      mask TypeOptions FP: Nominal | Abrupt | Incipient
      mask Display: image(imread('R.png','BackgroundColor',[1 1 1]))
  Se : Subsystem  mask{FP=Nominal, FT=0, FM=0}
      mask prompts: FP "Fault Profile:"; FT "Fault Injection Time:"; FM "Fault Magnitude/Slope/Bias/StuckAtValue"
      mask TypeOptions FP: Nominal | Abrupt | Incipient | Bias | StuckAt
      mask Display: image(imread('MSe.png','BackgroundColor',[1 1 1]))
  ZeroJunction : Subsystem
      mask Display: image(imread('0.png','BackgroundColor',[1 1 1]))
  Output : Outport
  wires:
  Se/1(E) -> OneJunction/1(u(1))
  OneJunction/1(y(1)) -> Se/1(F)
  OneJunction/2(y(2)) -> ZeroJunction/1(u(1))
  ZeroJunction/1(y(1)) -> OneJunction/2(u(2))
  OneJunction/3(y(3)) -> R/1(In)
  R/1(Out) -> OneJunction/3(u(3))
  ZeroJunction/2(y(2)) -> C/1(F)
  C/1(E) -> ZeroJunction/2(u(2))
  Function/1(y(1)) -> Se/2(Se)
  Input/1 -> Function/1(u(1))
  ZeroJunction/3(O) -> Output/1

== system VirtualADAPT/VirtualADAPTv1/PowerDistribution/CircuitBreakerEY280/ParallelConn ==
  u(1) : Inport
  u(2) : Inport  Port=2
  u(3) : Inport  Port=3
  u(4) : Inport  Port=4
  u(5) : Inport  Port=5
  Adder : Sum  Inputs=--  SaturateOnIntegerOverflow=on  InputSameDT=on  OutDataTypeStr=Inherit: Same as first input
  Gain : Gain  Gain=-1  SaturateOnIntegerOverflow=on  OutDataTypeStr=Inherit: Same as input  ParamDataTypeStr=Inherit: Same as input
  Mux : Mux  Inputs=5
  OffGuard : Fcn  Expr=(u(4) || !u(5))
  OnGuard : Fcn  Expr=(0)
  Product1 : Product  SaturateOnIntegerOverflow=on  RndMeth=Zero  InputSameDT=on  OutDataTypeStr=Inherit: Same as first input
  Product2 : Product  SaturateOnIntegerOverflow=on  RndMeth=Zero  InputSameDT=on  OutDataTypeStr=Inherit: Same as first input
  determineState : Subsystem
  y(1) : Outport
  y(2) : Outport  Port=2
  y(3) : Outport  Port=3
  O : Outport  Port=4
  State : Outport  Port=5
  wires:
  Adder/1 -> Gain/1
  u(4)/1 -> Mux/4
  u(5)/1 -> Mux/5
  Mux/1 -> OnGuard/1, OffGuard/1
  OnGuard/1 -> determineState/1(In1)
  OffGuard/1 -> determineState/2(In2)
  u(1)/1 -> Product1/1
  determineState/1(Out1) -> Product1/2, Product2/2, State/1
  Product1/1 -> O/1, y(2)/1, y(3)/1
  Gain/1 -> Product2/1
  Product2/1 -> y(1)/1
  u(2)/1 -> Adder/1
  u(3)/1 -> Adder/2
